simulink model "ospfb_2x_64kc_8i_16o"  (R2021a)
settings: Solver=VariableStepAuto  StartTime=0.0  StopTime=2304  RelTol=1e-3
undefined symbols (not defined in the file or in its folder): pfb_coeff_gen_calc
libraries: xbsIndex_r4 x37193, casper_library_misc x226, casper_library_delays x184
(part 197 of 313)

== system ospfb_2x_64kc_8i_16o/fft2/fft_direct/butterfly3_5/twiddle/coeff_gen/cosin ==
  theta : Inport
  misci : Inport  Port=2
  Constant4 : xbsIndex_r4/Constant  const=0  gui_display_data_type=Fixed-point  arith_type=Unsigned  n_bits=1  bin_pt=0  preci_type=Single  exp_width=8  frac_width=24  explicit_period=on  period=1  equ=P=C  opselect=C  inp2=PCIN>>17  opr=+  inp1=P  carry=CIN  dbl_ovrd=off  block_type=constant
  Constant5 : xbsIndex_r4/Constant  const=0  gui_display_data_type=Fixed-point  arith_type=Unsigned  n_bits=1  bin_pt=0  preci_type=Single  exp_width=8  frac_width=24  explicit_period=on  period=1  equ=P=C  opselect=C  inp2=PCIN>>17  opr=+  inp1=P  carry=CIN  dbl_ovrd=off  block_type=constant
  Delay : xbsIndex_r4/Delay  rst=off  infoeditControl=Selection of Reset will increase slice count due to use of real FFs and instead of SRLs  en=off  latency=bram_latency  reg_retiming=on  dbl_ovrd=off  block_type=delay
  Delay10 : xbsIndex_r4/Delay  rst=off  infoeditControl=Selection of Reset will increase slice count due to use of real FFs and instead of SRLs  en=off  latency=bram_latency  reg_retiming=on  dbl_ovrd=off  block_type=delay
  Delay8 : xbsIndex_r4/Delay  rst=off  infoeditControl=Selection of Reset will increase slice count due to use of real FFs and instead of SRLs  en=off  latency=bram_latency  reg_retiming=on  dbl_ovrd=off  block_type=delay
  ROM : xbsIndex_r4/ROM  depth=2^(12)  initVector=[55570736889 55567591166 55564707587 55561561864 55558416141 55555532561 55552386838 55549503259 55546357536 55543211813 55540328233 55537182510 55534298931 55531153208 55528007485 55525123906 55521978182 55519094603 55515948880 55512803157 55509919578 55506773854 55503890275 55500744552 55497598829 55494715250 55491569527 55488685948 55485540224 55482394501 55479510922 55476365199 55473481620 55470335897 55467190173 55464306594 55461160871 55458277292 55455131569 55451985846 55449102267 55445956543 55443072964 55439927241 55437043662 55433897939 55430752216 55427868637 55424722913 55421839334 55418693611 55415547888 55412664309 55409518586 55406635007 55403489283 55400343560 55397459981 55394314258 55391430679 55388284956 55385401377 55382255654 55379109930 55376226351 55373080628 55370197049 55367051326 55364167747 55361022024 55357876301 55354992721 55351846998 55348963419 55345817696 55342671973 55339788394 55336642671 55333759092 55330613369 55327729790 55324584066 55321438343 55318554764 55315409041 55312525462 55309379739 55306496160 55303350437 55300204714 55297321135 55294175411 55291291832 55288146109 55285262530 55282116807 55279233228 55276087505 55272941782 55270058203 55266912480 55264028901 55260883178 55257999598 55254853875 55251708152 55248824573 55245678850 55242795271 55239649548 55236765969 55233620246 55230736667 55227590944 55224445221 55221561642 55218415919 55215532340 55212386616 55209503037 55206357314 55203473735 55200328012 55197182289 55194298710 55191152987 55188269408 55185123685 55182240106 55179094383 55176210804 55173065081 55169919358 55167035779 55163890056 55161006477 55157860754 55154977175 55151831451 55148947872 55145802149 55142918570 55139772847 55136627124 55133743545 55130597822 55127714243 55124568520 55121684941 55118539218 55115655639 55112509916 55109626337 55106480614 55103597035 55100451312 55097305589 55094422010 55091276287 55088392708 55085246985 55082363406 55079217683 55076334104 55073188381 55070304802 55067159079 55064275500 55061129777 55058246198 55055100475 55051954752 55049071173 55045925450 55043041871 55039896148 55037012569 55033866846 55030983267 55027837544 55024953965 55021808242 55018924663 55015778940 55012895361 55009749638 55006866059 55003720336 55000836757 54997691034 54994545311 54991661732 54988516009 54985632430 54982486707 54979603128 54976457405 54973573826 54970428103 54967544524 54964398801 54961515222 54958369499 54955485920 54952340197 54949456618 54946310895 54943427316 54940281594 54937398015 54934252292 54931368713 54928222990 54925339411 54922193688 54919310109 54916164386 54913280807 54910135084 54907251505 54904105782 54901222203 54898076480 54895192901 54892047178 54889163599 54886017876 54883134297 54879988575 54877104996 54873959273 54871075694 54867929971 54865046392 54861900669 54859017090 54855871367 54852987788 54849842065 54846958486 54843812763 54840929184 54837783462 54834899883 54831754160 54828870581 54825724858 54822841279 54819695556 54816811977 54813666254 54810782675 54807636952 54804753373 54801607651 54798724072 54795578349 54792694770 54789549047 54786665468 54783519745 54780636166 54777490443 54774606864 54771461141 54768577563 54765431840 54762548261 54759402538 54756518959 54753373236 54750489657 54747343934 54744460355 54741576777 54738431054 54735547475 54732401752 54729518173 54726372450 54723488871 54720343148 54717459569 54714313847 54711430268 54708284545 54705400966 54702255243 54699371664 54696225941 54693342362 54690196640 54687313061 54684167338 54681283759 54678400180 54675254457 54672370878 54669225156 54666341577 54663195854 54660312275 54657166552 54654282973 54651137250 54648253672 54645107949 54642224370 54639078647 54636195068 54633311489 54630165766 54627282188 54624136465 54621252886 54618107163 54615223584 54612077861 54609194283 54606048560 54603164981 54600281402 54597135679 54594252100 54591106378 54588222799 54585077076 54582193497 54579047774 54576164195 54573018473 54570134894 54567251315 ...(+45156 chars)]  distributed_mem=Block RAM  rst=off  init_reg=0  en=off  latency=bram_latency  gui_display_data_type=Fixed-point  arith_type=Unsigned  n_bits=n_bits*2  bin_pt=0  preci_type=Single  exp_width=8  frac_width=24  optimize=Speed  use_rpm=on  dbl_ovrd=off  simulation_type=fast_sim  block_type=sprom
  Terminator : Terminator
  Terminator1 : Terminator
  Terminator4 : Terminator
  add_convert0 : Subsystem
  add_convert1 : Subsystem
  assert : xbsIndex_r4/Assert  assert_type=on  type_source=Explicitly  gui_display_data_type=Fixed-point  arith_type=Unsigned  n_bits=12  bin_pt=0  preci_type=Single  exp_width=8  frac_width=24  assert_rate=off  rate_source=Explicitly  period=1  output_port=on  block_type=assert
  c_to_ri : casper_library_misc/c_to_ri  n_bits=n_bits  bin_pt=bin_pt
      settings: SourceProductName=CASPER DSP Blockset
  invert0 : Subsystem  mask{n_bits=n_bits, bin_pt=bin_pt, neg_latency=neg_latency, mux_latency=mux_latency}
      mask prompts: n_bits "bit width"; bin_pt "binary point"; neg_latency "negate latency"; mux_latency "mux latency"
  invert1 : Subsystem  mask{n_bits=n_bits, bin_pt=bin_pt, neg_latency=neg_latency, mux_latency=mux_latency}
      mask prompts: n_bits "bit width"; bin_pt "binary point"; neg_latency "negate latency"; mux_latency "mux latency"
  -sin : Outport
  -cos : Outport  Port=2
  misco : Outport  Port=3
  wires:
  theta/1 -> assert/1
  assert/1 -> add_convert0/1(theta), add_convert1/1(theta)
  Constant4/1 -> add_convert0/2(misci)
  add_convert0/3(misco) -> Terminator/1
  misci/1 -> add_convert1/2(misci)
  add_convert1/3(misco) -> Delay/1
  add_convert0/2(add) -> ROM/1
  add_convert1/2(add) -> Terminator4/1
  ROM/1 -> c_to_ri/1
  add_convert1/1(negate) -> Delay8/1
  add_convert0/1(negate) -> Delay10/1
  Delay10/1 -> invert0/1(negate)
  Constant5/1 -> invert0/3(misci)
  c_to_ri/1 -> invert0/2(in)
  Delay8/1 -> invert1/1(negate)
  c_to_ri/2 -> invert1/2(in)
  invert0/2(misco) -> Terminator1/1
  Delay/1 -> invert1/3(misci)
  invert0/1(out) -> -sin/1
  invert1/1(out) -> -cos/1
  invert1/2(misco) -> misco/1
